simulink model "Demo_TISBeamShapes_cs"  (Simulink 7.6) (continued)
== system Demo_TISBeamShapes_cs == (continued)
      settings: DeleteFcn=PreScanBlockDeleteFcn;
      mask Help: eval('openHelp(222)')
      mask Display: image(imread('State.png'))
  STATE_Sphere_66 : Subsystem  Tag=State  Priority=0  mask(PreScan Sink Block: State){x0=20, y0=3, z0=4, Roll0=0, Pitch0=0, Yaw0=0, TimeStep=1/20}
      mask prompts: x0 "Ini x-position VEHICLE wrt GLOBAL axis system [m]"; y0 "Ini y-position VEHICLE wrt GLOBAL axis system [m]"; z0 "Ini z-position VEHICLE wrt GLOBAL axis system [m]"; Roll0 "Ini roll-angle BODY wrt VEHICLE axis system [deg]"; Pitch0 "Ini pitch-angle BODY wrt VEHICLE axis system [deg]"; Yaw0 "Ini yaw-angle VEHICLE wrt GLOBAL axis system [deg]"; TimeStep "Time Step [s]"
      mask help:
      | The Actor State contains a vector signal of size (9x1), containing the following signals:
      |
      | -  Position                  (x,y,z)                                     [m]
      | -  Velocity                  (vx,vy,vz)                               [m/s]
      | -  Angles                    (Roll, Pitch, Yaw)                   [rad]
      |
      | The Actor state is defined in the global axis system.
      | The state is superponed on the Initial position defined in the GUI.
      |
      | For more information, please click Help.
      settings: DeleteFcn=PreScanBlockDeleteFcn;
      mask Help: eval('openHelp(222)')
      mask Display: image(imread('State.png'))
  STATE_Sphere_67 : Subsystem  Tag=State  Priority=0  mask(PreScan Sink Block: State){x0=20, y0=3, z0=5, Roll0=0, Pitch0=0, Yaw0=0, TimeStep=1/20}
      mask prompts: x0 "Ini x-position VEHICLE wrt GLOBAL axis system [m]"; y0 "Ini y-position VEHICLE wrt GLOBAL axis system [m]"; z0 "Ini z-position VEHICLE wrt GLOBAL axis system [m]"; Roll0 "Ini roll-angle BODY wrt VEHICLE axis system [deg]"; Pitch0 "Ini pitch-angle BODY wrt VEHICLE axis system [deg]"; Yaw0 "Ini yaw-angle VEHICLE wrt GLOBAL axis system [deg]"; TimeStep "Time Step [s]"
      mask help:
      | The Actor State contains a vector signal of size (9x1), containing the following signals:
      |
      | -  Position                  (x,y,z)                                     [m]
      | -  Velocity                  (vx,vy,vz)                               [m/s]
      | -  Angles                    (Roll, Pitch, Yaw)                   [rad]
      |
      | The Actor state is defined in the global axis system.
      | The state is superponed on the Initial position defined in the GUI.
      |
      | For more information, please click Help.
      settings: DeleteFcn=PreScanBlockDeleteFcn;
      mask Help: eval('openHelp(222)')
      mask Display: image(imread('State.png'))
  STATE_Sphere_68 : Subsystem  Tag=State  Priority=0  mask(PreScan Sink Block: State){x0=20, y0=3, z0=6, Roll0=0, Pitch0=0, Yaw0=0, TimeStep=1/20}
      mask prompts: x0 "Ini x-position VEHICLE wrt GLOBAL axis system [m]"; y0 "Ini y-position VEHICLE wrt GLOBAL axis system [m]"; z0 "Ini z-position VEHICLE wrt GLOBAL axis system [m]"; Roll0 "Ini roll-angle BODY wrt VEHICLE axis system [deg]"; Pitch0 "Ini pitch-angle BODY wrt VEHICLE axis system [deg]"; Yaw0 "Ini yaw-angle VEHICLE wrt GLOBAL axis system [deg]"; TimeStep "Time Step [s]"
      mask help:
      | The Actor State contains a vector signal of size (9x1), containing the following signals:
      |
      | -  Position                  (x,y,z)                                     [m]
      | -  Velocity                  (vx,vy,vz)                               [m/s]
      | -  Angles                    (Roll, Pitch, Yaw)                   [rad]
      |
      | The Actor state is defined in the global axis system.
      | The state is superponed on the Initial position defined in the GUI.
      |
      | For more information, please click Help.
      settings: DeleteFcn=PreScanBlockDeleteFcn;
      mask Help: eval('openHelp(222)')
      mask Display: image(imread('State.png'))
  STATE_Sphere_69 : Subsystem  Tag=State  Priority=0  mask(PreScan Sink Block: State){x0=20, y0=3, z0=7, Roll0=0, Pitch0=0, Yaw0=0, TimeStep=1/20}
      mask prompts: x0 "Ini x-position VEHICLE wrt GLOBAL axis system [m]"; y0 "Ini y-position VEHICLE wrt GLOBAL axis system [m]"; z0 "Ini z-position VEHICLE wrt GLOBAL axis system [m]"; Roll0 "Ini roll-angle BODY wrt VEHICLE axis system [deg]"; Pitch0 "Ini pitch-angle BODY wrt VEHICLE axis system [deg]"; Yaw0 "Ini yaw-angle VEHICLE wrt GLOBAL axis system [deg]"; TimeStep "Time Step [s]"
      mask help:
      | The Actor State contains a vector signal of size (9x1), containing the following signals:
      |
      | -  Position                  (x,y,z)                                     [m]
      | -  Velocity                  (vx,vy,vz)                               [m/s]
      | -  Angles                    (Roll, Pitch, Yaw)                   [rad]
      |
      | The Actor state is defined in the global axis system.
      | The state is superponed on the Initial position defined in the GUI.
      |
      | For more information, please click Help.
      settings: DeleteFcn=PreScanBlockDeleteFcn;
      mask Help: eval('openHelp(222)')
      mask Display: image(imread('State.png'))
  STATE_Sphere_7 : Subsystem  Tag=State  Priority=0  mask(PreScan Sink Block: State){x0=20, y0=-4, z0=8, Roll0=0, Pitch0=0, Yaw0=0, TimeStep=1/20}
      mask prompts: x0 "Ini x-position VEHICLE wrt GLOBAL axis system [m]"; y0 "Ini y-position VEHICLE wrt GLOBAL axis system [m]"; z0 "Ini z-position VEHICLE wrt GLOBAL axis system [m]"; Roll0 "Ini roll-angle BODY wrt VEHICLE axis system [deg]"; Pitch0 "Ini pitch-angle BODY wrt VEHICLE axis system [deg]"; Yaw0 "Ini yaw-angle VEHICLE wrt GLOBAL axis system [deg]"; TimeStep "Time Step [s]"
      mask help:
      | The Actor State contains a vector signal of size (9x1), containing the following signals:
      |
      | -  Position                  (x,y,z)                                     [m]
      | -  Velocity                  (vx,vy,vz)                               [m/s]
      | -  Angles                    (Roll, Pitch, Yaw)                   [rad]
      |
      | The Actor state is defined in the global axis system.
      | The state is superponed on the Initial position defined in the GUI.
      |
      | For more information, please click Help.
      settings: DeleteFcn=PreScanBlockDeleteFcn;
      mask Help: eval('openHelp(222)')
      mask Display: image(imread('State.png'))
  STATE_Sphere_70 : Subsystem  Tag=State  Priority=0  mask(PreScan Sink Block: State){x0=20, y0=3, z0=8, Roll0=0, Pitch0=0, Yaw0=0, TimeStep=1/20}
      mask prompts: x0 "Ini x-position VEHICLE wrt GLOBAL axis system [m]"; y0 "Ini y-position VEHICLE wrt GLOBAL axis system [m]"; z0 "Ini z-position VEHICLE wrt GLOBAL axis system [m]"; Roll0 "Ini roll-angle BODY wrt VEHICLE axis system [deg]"; Pitch0 "Ini pitch-angle BODY wrt VEHICLE axis system [deg]"; Yaw0 "Ini yaw-angle VEHICLE wrt GLOBAL axis system [deg]"; TimeStep "Time Step [s]"
      mask help:
      | The Actor State contains a vector signal of size (9x1), containing the following signals:
      |
      | -  Position                  (x,y,z)                                     [m]
      | -  Velocity                  (vx,vy,vz)                               [m/s]
      | -  Angles                    (Roll, Pitch, Yaw)                   [rad]
      |
      | The Actor state is defined in the global axis system.
      | The state is superponed on the Initial position defined in the GUI.
      |
      | For more information, please click Help.
      settings: DeleteFcn=PreScanBlockDeleteFcn;
      mask Help: eval('openHelp(222)')
      mask Display: image(imread('State.png'))
  STATE_Sphere_71 : Subsystem  Tag=State  Priority=0  mask(PreScan Sink Block: State){x0=20, y0=3, z0=9, Roll0=0, Pitch0=0, Yaw0=0, TimeStep=1/20}
      mask prompts: x0 "Ini x-position VEHICLE wrt GLOBAL axis system [m]"; y0 "Ini y-position VEHICLE wrt GLOBAL axis system [m]"; z0 "Ini z-position VEHICLE wrt GLOBAL axis system [m]"; Roll0 "Ini roll-angle BODY wrt VEHICLE axis system [deg]"; Pitch0 "Ini pitch-angle BODY wrt VEHICLE axis system [deg]"; Yaw0 "Ini yaw-angle VEHICLE wrt GLOBAL axis system [deg]"; TimeStep "Time Step [s]"
      mask help:
      | The Actor State contains a vector signal of size (9x1), containing the following signals:
      |
      | -  Position                  (x,y,z)                                     [m]
      | -  Velocity                  (vx,vy,vz)                               [m/s]
      | -  Angles                    (Roll, Pitch, Yaw)                   [rad]
      |
      | The Actor state is defined in the global axis system.
      | The state is superponed on the Initial position defined in the GUI.
      |
      | For more information, please click Help.
      settings: DeleteFcn=PreScanBlockDeleteFcn;
      mask Help: eval('openHelp(222)')
      mask Display: image(imread('State.png'))
  STATE_Sphere_72 : Subsystem  Tag=State  Priority=0  mask(PreScan Sink Block: State){x0=20, y0=3, z0=10, Roll0=0, Pitch0=0, Yaw0=0, TimeStep=1/20}
      mask prompts: x0 "Ini x-position VEHICLE wrt GLOBAL axis system [m]"; y0 "Ini y-position VEHICLE wrt GLOBAL axis system [m]"; z0 "Ini z-position VEHICLE wrt GLOBAL axis system [m]"; Roll0 "Ini roll-angle BODY wrt VEHICLE axis system [deg]"; Pitch0 "Ini pitch-angle BODY wrt VEHICLE axis system [deg]"; Yaw0 "Ini yaw-angle VEHICLE wrt GLOBAL axis system [deg]"; TimeStep "Time Step [s]"
      mask help:
      | The Actor State contains a vector signal of size (9x1), containing the following signals:
      |
      | -  Position                  (x,y,z)                                     [m]
      | -  Velocity                  (vx,vy,vz)                               [m/s]
      | -  Angles                    (Roll, Pitch, Yaw)                   [rad]
      |
      | The Actor state is defined in the global axis system.
      | The state is superponed on the Initial position defined in the GUI.
      |
      | For more information, please click Help.
      settings: DeleteFcn=PreScanBlockDeleteFcn;
      mask Help: eval('openHelp(222)')
      mask Display: image(imread('State.png'))
  STATE_Sphere_73 : Subsystem  Tag=State  Priority=0  mask(PreScan Sink Block: State){x0=20, y0=4, z0=2, Roll0=0, Pitch0=0, Yaw0=0, TimeStep=1/20}
      mask prompts: x0 "Ini x-position VEHICLE wrt GLOBAL axis system [m]"; y0 "Ini y-position VEHICLE wrt GLOBAL axis system [m]"; z0 "Ini z-position VEHICLE wrt GLOBAL axis system [m]"; Roll0 "Ini roll-angle BODY wrt VEHICLE axis system [deg]"; Pitch0 "Ini pitch-angle BODY wrt VEHICLE axis system [deg]"; Yaw0 "Ini yaw-angle VEHICLE wrt GLOBAL axis system [deg]"; TimeStep "Time Step [s]"
      mask help:
      | The Actor State contains a vector signal of size (9x1), containing the following signals:
      |
      | -  Position                  (x,y,z)                                     [m]
      | -  Velocity                  (vx,vy,vz)                               [m/s]
      | -  Angles                    (Roll, Pitch, Yaw)                   [rad]
      |
      | The Actor state is defined in the global axis system.
      | The state is superponed on the Initial position defined in the GUI.
      |
      | For more information, please click Help.
      settings: DeleteFcn=PreScanBlockDeleteFcn;
      mask Help: eval('openHelp(222)')
      mask Display: image(imread('State.png'))
  STATE_Sphere_74 : Subsystem  Tag=State  Priority=0  mask(PreScan Sink Block: State){x0=20, y0=4, z0=3, Roll0=0, Pitch0=0, Yaw0=0, TimeStep=1/20}
      mask prompts: x0 "Ini x-position VEHICLE wrt GLOBAL axis system [m]"; y0 "Ini y-position VEHICLE wrt GLOBAL axis system [m]"; z0 "Ini z-position VEHICLE wrt GLOBAL axis system [m]"; Roll0 "Ini roll-angle BODY wrt VEHICLE axis system [deg]"; Pitch0 "Ini pitch-angle BODY wrt VEHICLE axis system [deg]"; Yaw0 "Ini yaw-angle VEHICLE wrt GLOBAL axis system [deg]"; TimeStep "Time Step [s]"
      mask help:
      | The Actor State contains a vector signal of size (9x1), containing the following signals:
      |
      | -  Position                  (x,y,z)                                     [m]
      | -  Velocity                  (vx,vy,vz)                               [m/s]
      | -  Angles                    (Roll, Pitch, Yaw)                   [rad]
      |
      | The Actor state is defined in the global axis system.
      | The state is superponed on the Initial position defined in the GUI.
      |
      | For more information, please click Help.
      settings: DeleteFcn=PreScanBlockDeleteFcn;
      mask Help: eval('openHelp(222)')
      mask Display: image(imread('State.png'))
  STATE_Sphere_75 : Subsystem  Tag=State  Priority=0  mask(PreScan Sink Block: State){x0=20, y0=4, z0=4, Roll0=0, Pitch0=0, Yaw0=0, TimeStep=1/20}
      mask prompts: x0 "Ini x-position VEHICLE wrt GLOBAL axis system [m]"; y0 "Ini y-position VEHICLE wrt GLOBAL axis system [m]"; z0 "Ini z-position VEHICLE wrt GLOBAL axis system [m]"; Roll0 "Ini roll-angle BODY wrt VEHICLE axis system [deg]"; Pitch0 "Ini pitch-angle BODY wrt VEHICLE axis system [deg]"; Yaw0 "Ini yaw-angle VEHICLE wrt GLOBAL axis system [deg]"; TimeStep "Time Step [s]"
      mask help:
      | The Actor State contains a vector signal of size (9x1), containing the following signals:
      |
      | -  Position                  (x,y,z)                                     [m]
      | -  Velocity                  (vx,vy,vz)                               [m/s]
      | -  Angles                    (Roll, Pitch, Yaw)                   [rad]
      |
      | The Actor state is defined in the global axis system.
      | The state is superponed on the Initial position defined in the GUI.
      |
      | For more information, please click Help.
      settings: DeleteFcn=PreScanBlockDeleteFcn;
      mask Help: eval('openHelp(222)')
      mask Display: image(imread('State.png'))
  STATE_Sphere_76 : Subsystem  Tag=State  Priority=0  mask(PreScan Sink Block: State){x0=20, y0=4, z0=5, Roll0=0, Pitch0=0, Yaw0=0, TimeStep=1/20}
      mask prompts: x0 "Ini x-position VEHICLE wrt GLOBAL axis system [m]"; y0 "Ini y-position VEHICLE wrt GLOBAL axis system [m]"; z0 "Ini z-position VEHICLE wrt GLOBAL axis system [m]"; Roll0 "Ini roll-angle BODY wrt VEHICLE axis system [deg]"; Pitch0 "Ini pitch-angle BODY wrt VEHICLE axis system [deg]"; Yaw0 "Ini yaw-angle VEHICLE wrt GLOBAL axis system [deg]"; TimeStep "Time Step [s]"
      mask help:
      | The Actor State contains a vector signal of size (9x1), containing the following signals:
      |
      | -  Position                  (x,y,z)                                     [m]
      | -  Velocity                  (vx,vy,vz)                               [m/s]
      | -  Angles                    (Roll, Pitch, Yaw)                   [rad]
      |
      | The Actor state is defined in the global axis system.
      | The state is superponed on the Initial position defined in the GUI.
      |
      | For more information, please click Help.
      settings: DeleteFcn=PreScanBlockDeleteFcn;
      mask Help: eval('openHelp(222)')
      mask Display: image(imread('State.png'))
  STATE_Sphere_77 : Subsystem  Tag=State  Priority=0  mask(PreScan Sink Block: State){x0=20, y0=4, z0=6, Roll0=0, Pitch0=0, Yaw0=0, TimeStep=1/20}
      mask prompts: x0 "Ini x-position VEHICLE wrt GLOBAL axis system [m]"; y0 "Ini y-position VEHICLE wrt GLOBAL axis system [m]"; z0 "Ini z-position VEHICLE wrt GLOBAL axis system [m]"; Roll0 "Ini roll-angle BODY wrt VEHICLE axis system [deg]"; Pitch0 "Ini pitch-angle BODY wrt VEHICLE axis system [deg]"; Yaw0 "Ini yaw-angle VEHICLE wrt GLOBAL axis system [deg]"; TimeStep "Time Step [s]"
      mask help:
      | The Actor State contains a vector signal of size (9x1), containing the following signals:
      |
      | -  Position                  (x,y,z)                                     [m]
      | -  Velocity                  (vx,vy,vz)                               [m/s]
      | -  Angles                    (Roll, Pitch, Yaw)                   [rad]
      |
      | The Actor state is defined in the global axis system.
      | The state is superponed on the Initial position defined in the GUI.
      |
      | For more information, please click Help.
      settings: DeleteFcn=PreScanBlockDeleteFcn;
      mask Help: eval('openHelp(222)')
      mask Display: image(imread('State.png'))
  STATE_Sphere_78 : Subsystem  Tag=State  Priority=0  mask(PreScan Sink Block: State){x0=20, y0=4, z0=7, Roll0=0, Pitch0=0, Yaw0=0, TimeStep=1/20}
      mask prompts: x0 "Ini x-position VEHICLE wrt GLOBAL axis system [m]"; y0 "Ini y-position VEHICLE wrt GLOBAL axis system [m]"; z0 "Ini z-position VEHICLE wrt GLOBAL axis system [m]"; Roll0 "Ini roll-angle BODY wrt VEHICLE axis system [deg]"; Pitch0 "Ini pitch-angle BODY wrt VEHICLE axis system [deg]"; Yaw0 "Ini yaw-angle VEHICLE wrt GLOBAL axis system [deg]"; TimeStep "Time Step [s]"
      mask help:
      | The Actor State contains a vector signal of size (9x1), containing the following signals:
      |
      | -  Position                  (x,y,z)                                     [m]
      | -  Velocity                  (vx,vy,vz)                               [m/s]
      | -  Angles                    (Roll, Pitch, Yaw)                   [rad]
      |
      | The Actor state is defined in the global axis system.
      | The state is superponed on the Initial position defined in the GUI.
      |
      | For more information, please click Help.
      settings: DeleteFcn=PreScanBlockDeleteFcn;
      mask Help: eval('openHelp(222)')
      mask Display: image(imread('State.png'))
  STATE_Sphere_79 : Subsystem  Tag=State  Priority=0  mask(PreScan Sink Block: State){x0=20, y0=4, z0=8, Roll0=0, Pitch0=0, Yaw0=0, TimeStep=1/20}
      mask prompts: x0 "Ini x-position VEHICLE wrt GLOBAL axis system [m]"; y0 "Ini y-position VEHICLE wrt GLOBAL axis system [m]"; z0 "Ini z-position VEHICLE wrt GLOBAL axis system [m]"; Roll0 "Ini roll-angle BODY wrt VEHICLE axis system [deg]"; Pitch0 "Ini pitch-angle BODY wrt VEHICLE axis system [deg]"; Yaw0 "Ini yaw-angle VEHICLE wrt GLOBAL axis system [deg]"; TimeStep "Time Step [s]"
      mask help:
      | The Actor State contains a vector signal of size (9x1), containing the following signals:
      |
      | -  Position                  (x,y,z)                                     [m]
      | -  Velocity                  (vx,vy,vz)                               [m/s]
      | -  Angles                    (Roll, Pitch, Yaw)                   [rad]
      |
      | The Actor state is defined in the global axis system.
      | The state is superponed on the Initial position defined in the GUI.
      |
      | For more information, please click Help.
      settings: DeleteFcn=PreScanBlockDeleteFcn;
      mask Help: eval('openHelp(222)')
      mask Display: image(imread('State.png'))
  STATE_Sphere_8 : Subsystem  Tag=State  Priority=0  mask(PreScan Sink Block: State){x0=20, y0=-4, z0=9, Roll0=0, Pitch0=0, Yaw0=0, TimeStep=1/20}
      mask prompts: x0 "Ini x-position VEHICLE wrt GLOBAL axis system [m]"; y0 "Ini y-position VEHICLE wrt GLOBAL axis system [m]"; z0 "Ini z-position VEHICLE wrt GLOBAL axis system [m]"; Roll0 "Ini roll-angle BODY wrt VEHICLE axis system [deg]"; Pitch0 "Ini pitch-angle BODY wrt VEHICLE axis system [deg]"; Yaw0 "Ini yaw-angle VEHICLE wrt GLOBAL axis system [deg]"; TimeStep "Time Step [s]"
      mask help:
      | The Actor State contains a vector signal of size (9x1), containing the following signals:
      |
      | -  Position                  (x,y,z)                                     [m]
      | -  Velocity                  (vx,vy,vz)                               [m/s]
      | -  Angles                    (Roll, Pitch, Yaw)                   [rad]
      |
      | The Actor state is defined in the global axis system.
      | The state is superponed on the Initial position defined in the GUI.
      |
      | For more information, please click Help.
      settings: DeleteFcn=PreScanBlockDeleteFcn;
      mask Help: eval('openHelp(222)')
      mask Display: image(imread('State.png'))
  STATE_Sphere_80 : Subsystem  Tag=State  Priority=0  mask(PreScan Sink Block: State){x0=20, y0=4, z0=9, Roll0=0, Pitch0=0, Yaw0=0, TimeStep=1/20}
      mask prompts: x0 "Ini x-position VEHICLE wrt GLOBAL axis system [m]"; y0 "Ini y-position VEHICLE wrt GLOBAL axis system [m]"; z0 "Ini z-position VEHICLE wrt GLOBAL axis system [m]"; Roll0 "Ini roll-angle BODY wrt VEHICLE axis system [deg]"; Pitch0 "Ini pitch-angle BODY wrt VEHICLE axis system [deg]"; Yaw0 "Ini yaw-angle VEHICLE wrt GLOBAL axis system [deg]"; TimeStep "Time Step [s]"
      mask help:
      | The Actor State contains a vector signal of size (9x1), containing the following signals:
      |
      | -  Position                  (x,y,z)                                     [m]
      | -  Velocity                  (vx,vy,vz)                               [m/s]
      | -  Angles                    (Roll, Pitch, Yaw)                   [rad]
      |
      | The Actor state is defined in the global axis system.
      | The state is superponed on the Initial position defined in the GUI.
      |
      | For more information, please click Help.
      settings: DeleteFcn=PreScanBlockDeleteFcn;
      mask Help: eval('openHelp(222)')
      mask Display: image(imread('State.png'))
  STATE_Sphere_81 : Subsystem  Tag=State  Priority=0  mask(PreScan Sink Block: State){x0=20, y0=4, z0=10, Roll0=0, Pitch0=0, Yaw0=0, TimeStep=1/20}
      mask prompts: x0 "Ini x-position VEHICLE wrt GLOBAL axis system [m]"; y0 "Ini y-position VEHICLE wrt GLOBAL axis system [m]"; z0 "Ini z-position VEHICLE wrt GLOBAL axis system [m]"; Roll0 "Ini roll-angle BODY wrt VEHICLE axis system [deg]"; Pitch0 "Ini pitch-angle BODY wrt VEHICLE axis system [deg]"; Yaw0 "Ini yaw-angle VEHICLE wrt GLOBAL axis system [deg]"; TimeStep "Time Step [s]"
      mask help:
      | The Actor State contains a vector signal of size (9x1), containing the following signals:
      |
      | -  Position                  (x,y,z)                                     [m]
      | -  Velocity                  (vx,vy,vz)                               [m/s]
      | -  Angles                    (Roll, Pitch, Yaw)                   [rad]
      |
      | The Actor state is defined in the global axis system.
      | The state is superponed on the Initial position defined in the GUI.
      |
      | For more information, please click Help.
      settings: DeleteFcn=PreScanBlockDeleteFcn;
      mask Help: eval('openHelp(222)')
      mask Display: image(imread('State.png'))
  STATE_Sphere_9 : Subsystem  Tag=State  Priority=0  mask(PreScan Sink Block: State){x0=20, y0=-4, z0=10, Roll0=0, Pitch0=0, Yaw0=0, TimeStep=1/20}
      mask prompts: x0 "Ini x-position VEHICLE wrt GLOBAL axis system [m]"; y0 "Ini y-position VEHICLE wrt GLOBAL axis system [m]"; z0 "Ini z-position VEHICLE wrt GLOBAL axis system [m]"; Roll0 "Ini roll-angle BODY wrt VEHICLE axis system [deg]"; Pitch0 "Ini pitch-angle BODY wrt VEHICLE axis system [deg]"; Yaw0 "Ini yaw-angle VEHICLE wrt GLOBAL axis system [deg]"; TimeStep "Time Step [s]"
      mask help:
      | The Actor State contains a vector signal of size (9x1), containing the following signals:
      |
      | -  Position                  (x,y,z)                                     [m]
      | -  Velocity                  (vx,vy,vz)                               [m/s]
      | -  Angles                    (Roll, Pitch, Yaw)                   [rad]
      |
      | The Actor state is defined in the global axis system.
      | The state is superponed on the Initial position defined in the GUI.
      |
      | For more information, please click Help.
      settings: DeleteFcn=PreScanBlockDeleteFcn;
      mask Help: eval('openHelp(222)')
      mask Display: image(imread('State.png'))
  SetActuators : Subsystem  Tag=SetActuators  Priority=1  mask(PreScan Simulation Core Block){TimeStep=1/20, NrOfActuators=1260}
      mask prompts: TimeStep "Timestep [s]"; NrOfActuators "Number of actuator values"
      mask help:
      | Please do not remove this block from the PreScan compilation sheet,
      | as it contains essential configuration parameters for a PreScan simulation.
      settings: DeleteFcn=PreScanBlockDeleteFcn;
  SimCoreConfiguration : M-S-Function  Tag=SimCoreConfiguration  Description=CAD8B8374CE53CE7DF3567A6B8CC3B00F170F6ABFE8D6F1F6CD897A2C413C5A6  FunctionName=SimCoreBlock  Parameters=ExperimentXML PortsXML SimulationCore HostName CheckStart EnableLog RfR EnableGPUAccel ShowComputationTime  mask(PreScan Simulation Core Configurator){SimulationCore=NUTS, HostName=0, CheckStart=0, prescanversion='7.4.0', EnableLog=off, RfR=0, EnableGPUAccel=off, ShowComputationTime=off}
      mask prompts: SimulationCore "Simulation Core"; HostName "Simulation Host"; CheckStart "Show START Dialog"; prescanversion "PreScan Version"; EnableLog "Create TA Log"; RfR "Record for Replay"; EnableGPUAccel "Enable GPU Acceleration"; ShowComputationTime "Show ComputationTime Scope"
      mask help:
      | Please do not remove this block from the PreScan compilation sheet,
      | as it contains essential configuration parameter for a PreScan simulation.
      mask init:
      | model = bdroot;
      | ExperimentXML = [model(1:end-3) '.pex'];
      | PortsXML = [model '_ports.pcd'];
      settings: DeleteFcn=PreScanBlockDeleteFcn;  ModelCloseFcn=assignin('base',['model_closed_' bdroot],true);
      mask Help: eval('openHelp(812)')
      mask Display: image(imread('prescanlogo.ico'))
  SimCoreInfo : Subsystem  Tag=SimCoreInfo
  Sphere_1 : Subsystem  Tag=WorldObjectSubSystem  InitFcn=UniqueId = '1001';
      mask Display: image(imread('Sphere.jpg'));color('white');text(0,0,' ID=1001\n')
  Sphere_10 : Subsystem  Tag=WorldObjectSubSystem  InitFcn=UniqueId = '1010';
      mask Display: image(imread('Sphere.jpg'));color('white');text(0,0,' ID=1010\n')
  Sphere_11 : Subsystem  Tag=WorldObjectSubSystem  InitFcn=UniqueId = '1011';
      mask Display: image(imread('Sphere.jpg'));color('white');text(0,0,' ID=1011\n')
  Sphere_12 : Subsystem  Tag=WorldObjectSubSystem  InitFcn=UniqueId = '1012';
      mask Display: image(imread('Sphere.jpg'));color('white');text(0,0,' ID=1012\n')
  Sphere_13 : Subsystem  Tag=WorldObjectSubSystem  InitFcn=UniqueId = '1013';
      mask Display: image(imread('Sphere.jpg'));color('white');text(0,0,' ID=1013\n')
  Sphere_14 : Subsystem  Tag=WorldObjectSubSystem  InitFcn=UniqueId = '1014';
      mask Display: image(imread('Sphere.jpg'));color('white');text(0,0,' ID=1014\n')
  Sphere_15 : Subsystem  Tag=WorldObjectSubSystem  InitFcn=UniqueId = '1015';
      mask Display: image(imread('Sphere.jpg'));color('white');text(0,0,' ID=1015\n')
  Sphere_16 : Subsystem  Tag=WorldObjectSubSystem  InitFcn=UniqueId = '1016';
      mask Display: image(imread('Sphere.jpg'));color('white');text(0,0,' ID=1016\n')
  Sphere_17 : Subsystem  Tag=WorldObjectSubSystem  InitFcn=UniqueId = '1017';
      mask Display: image(imread('Sphere.jpg'));color('white');text(0,0,' ID=1017\n')
  Sphere_18 : Subsystem  Tag=WorldObjectSubSystem  InitFcn=UniqueId = '1018';
      mask Display: image(imread('Sphere.jpg'));color('white');text(0,0,' ID=1018\n')
  Sphere_19 : Subsystem  Tag=WorldObjectSubSystem  InitFcn=UniqueId = '1019';
      mask Display: image(imread('Sphere.jpg'));color('white');text(0,0,' ID=1019\n')
  Sphere_2 : Subsystem  Tag=WorldObjectSubSystem  InitFcn=UniqueId = '1002';
      mask Display: image(imread('Sphere.jpg'));color('white');text(0,0,' ID=1002\n')
  Sphere_20 : Subsystem  Tag=WorldObjectSubSystem  InitFcn=UniqueId = '1020';
      mask Display: image(imread('Sphere.jpg'));color('white');text(0,0,' ID=1020\n')
  Sphere_21 : Subsystem  Tag=WorldObjectSubSystem  InitFcn=UniqueId = '1021';
      mask Display: image(imread('Sphere.jpg'));color('white');text(0,0,' ID=1021\n')
  Sphere_22 : Subsystem  Tag=WorldObjectSubSystem  InitFcn=UniqueId = '1022';
      mask Display: image(imread('Sphere.jpg'));color('white');text(0,0,' ID=1022\n')
  Sphere_23 : Subsystem  Tag=WorldObjectSubSystem  InitFcn=UniqueId = '1023';
      mask Display: image(imread('Sphere.jpg'));color('white');text(0,0,' ID=1023\n')
  Sphere_24 : Subsystem  Tag=WorldObjectSubSystem  InitFcn=UniqueId = '1024';
      mask Display: image(imread('Sphere.jpg'));color('white');text(0,0,' ID=1024\n')
  Sphere_25 : Subsystem  Tag=WorldObjectSubSystem  InitFcn=UniqueId = '1025';
      mask Display: image(imread('Sphere.jpg'));color('white');text(0,0,' ID=1025\n')
  Sphere_26 : Subsystem  Tag=WorldObjectSubSystem  InitFcn=UniqueId = '1026';
      mask Display: image(imread('Sphere.jpg'));color('white');text(0,0,' ID=1026\n')
  Sphere_27 : Subsystem  Tag=WorldObjectSubSystem  InitFcn=UniqueId = '1027';
      mask Display: image(imread('Sphere.jpg'));color('white');text(0,0,' ID=1027\n')
  Sphere_28 : Subsystem  Tag=WorldObjectSubSystem  InitFcn=UniqueId = '1028';
      mask Display: image(imread('Sphere.jpg'));color('white');text(0,0,' ID=1028\n')
  Sphere_29 : Subsystem  Tag=WorldObjectSubSystem  InitFcn=UniqueId = '1029';
      mask Display: image(imread('Sphere.jpg'));color('white');text(0,0,' ID=1029\n')
  Sphere_3 : Subsystem  Tag=WorldObjectSubSystem  InitFcn=UniqueId = '1003';
      mask Display: image(imread('Sphere.jpg'));color('white');text(0,0,' ID=1003\n')
  Sphere_30 : Subsystem  Tag=WorldObjectSubSystem  InitFcn=UniqueId = '1030';
      mask Display: image(imread('Sphere.jpg'));color('white');text(0,0,' ID=1030\n')
  Sphere_31 : Subsystem  Tag=WorldObjectSubSystem  InitFcn=UniqueId = '1031';
      mask Display: image(imread('Sphere.jpg'));color('white');text(0,0,' ID=1031\n')
  Sphere_32 : Subsystem  Tag=WorldObjectSubSystem  InitFcn=UniqueId = '1032';
      mask Display: image(imread('Sphere.jpg'));color('white');text(0,0,' ID=1032\n')
  Sphere_33 : Subsystem  Tag=WorldObjectSubSystem  InitFcn=UniqueId = '1033';
      mask Display: image(imread('Sphere.jpg'));color('white');text(0,0,' ID=1033\n')
  Sphere_34 : Subsystem  Tag=WorldObjectSubSystem  InitFcn=UniqueId = '1034';
      mask Display: image(imread('Sphere.jpg'));color('white');text(0,0,' ID=1034\n')
  Sphere_35 : Subsystem  Tag=WorldObjectSubSystem  InitFcn=UniqueId = '1035';
      mask Display: image(imread('Sphere.jpg'));color('white');text(0,0,' ID=1035\n')
  Sphere_36 : Subsystem  Tag=WorldObjectSubSystem  InitFcn=UniqueId = '1036';
      mask Display: image(imread('Sphere.jpg'));color('white');text(0,0,' ID=1036\n')
  Sphere_37 : Subsystem  Tag=WorldObjectSubSystem  InitFcn=UniqueId = '1037';
      mask Display: image(imread('Sphere.jpg'));color('white');text(0,0,' ID=1037\n')
  Sphere_38 : Subsystem  Tag=WorldObjectSubSystem  InitFcn=UniqueId = '1038';
      mask Display: image(imread('Sphere.jpg'));color('white');text(0,0,' ID=1038\n')
  Sphere_39 : Subsystem  Tag=WorldObjectSubSystem  InitFcn=UniqueId = '1039';
      mask Display: image(imread('Sphere.jpg'));color('white');text(0,0,' ID=1039\n')
  Sphere_4 : Subsystem  Tag=WorldObjectSubSystem  InitFcn=UniqueId = '1004';
      mask Display: image(imread('Sphere.jpg'));color('white');text(0,0,' ID=1004\n')
  Sphere_40 : Subsystem  Tag=WorldObjectSubSystem  InitFcn=UniqueId = '1040';
      mask Display: image(imread('Sphere.jpg'));color('white');text(0,0,' ID=1040\n')
  Sphere_41 : Subsystem  Tag=WorldObjectSubSystem  InitFcn=UniqueId = '1041';
      mask Display: image(imread('Sphere.jpg'));color('white');text(0,0,' ID=1041\n')
  Sphere_42 : Subsystem  Tag=WorldObjectSubSystem  InitFcn=UniqueId = '1042';
      mask Display: image(imread('Sphere.jpg'));color('white');text(0,0,' ID=1042\n')
  Sphere_43 : Subsystem  Tag=WorldObjectSubSystem  InitFcn=UniqueId = '1043';
      mask Display: image(imread('Sphere.jpg'));color('white');text(0,0,' ID=1043\n')
  Sphere_44 : Subsystem  Tag=WorldObjectSubSystem  InitFcn=UniqueId = '1044';
      mask Display: image(imread('Sphere.jpg'));color('white');text(0,0,' ID=1044\n')
  Sphere_45 : Subsystem  Tag=WorldObjectSubSystem  InitFcn=UniqueId = '1045';
      mask Display: image(imread('Sphere.jpg'));color('white');text(0,0,' ID=1045\n')
  Sphere_46 : Subsystem  Tag=WorldObjectSubSystem  InitFcn=UniqueId = '1046';
      mask Display: image(imread('Sphere.jpg'));color('white');text(0,0,' ID=1046\n')
  Sphere_47 : Subsystem  Tag=WorldObjectSubSystem  InitFcn=UniqueId = '1047';
      mask Display: image(imread('Sphere.jpg'));color('white');text(0,0,' ID=1047\n')
  Sphere_48 : Subsystem  Tag=WorldObjectSubSystem  InitFcn=UniqueId = '1048';
      mask Display: image(imread('Sphere.jpg'));color('white');text(0,0,' ID=1048\n')
  Sphere_49 : Subsystem  Tag=WorldObjectSubSystem  InitFcn=UniqueId = '1049';
      mask Display: image(imread('Sphere.jpg'));color('white');text(0,0,' ID=1049\n')
  Sphere_5 : Subsystem  Tag=WorldObjectSubSystem  InitFcn=UniqueId = '1005';
      mask Display: image(imread('Sphere.jpg'));color('white');text(0,0,' ID=1005\n')
  Sphere_50 : Subsystem  Tag=WorldObjectSubSystem  InitFcn=UniqueId = '1050';
      mask Display: image(imread('Sphere.jpg'));color('white');text(0,0,' ID=1050\n')
  Sphere_51 : Subsystem  Tag=WorldObjectSubSystem  InitFcn=UniqueId = '1051';
      mask Display: image(imread('Sphere.jpg'));color('white');text(0,0,' ID=1051\n')
  Sphere_52 : Subsystem  Tag=WorldObjectSubSystem  InitFcn=UniqueId = '1052';
      mask Display: image(imread('Sphere.jpg'));color('white');text(0,0,' ID=1052\n')
  Sphere_53 : Subsystem  Tag=WorldObjectSubSystem  InitFcn=UniqueId = '1053';
      mask Display: image(imread('Sphere.jpg'));color('white');text(0,0,' ID=1053\n')
  Sphere_54 : Subsystem  Tag=WorldObjectSubSystem  InitFcn=UniqueId = '1054';
      mask Display: image(imread('Sphere.jpg'));color('white');text(0,0,' ID=1054\n')
  Sphere_55 : Subsystem  Tag=WorldObjectSubSystem  InitFcn=UniqueId = '1055';
      mask Display: image(imread('Sphere.jpg'));color('white');text(0,0,' ID=1055\n')
  Sphere_56 : Subsystem  Tag=WorldObjectSubSystem  InitFcn=UniqueId = '1056';
      mask Display: image(imread('Sphere.jpg'));color('white');text(0,0,' ID=1056\n')
  Sphere_57 : Subsystem  Tag=WorldObjectSubSystem  InitFcn=UniqueId = '1057';
      mask Display: image(imread('Sphere.jpg'));color('white');text(0,0,' ID=1057\n')
  Sphere_58 : Subsystem  Tag=WorldObjectSubSystem  InitFcn=UniqueId = '1058';
      mask Display: image(imread('Sphere.jpg'));color('white');text(0,0,' ID=1058\n')
  Sphere_59 : Subsystem  Tag=WorldObjectSubSystem  InitFcn=UniqueId = '1059';
      mask Display: image(imread('Sphere.jpg'));color('white');text(0,0,' ID=1059\n')
  Sphere_6 : Subsystem  Tag=WorldObjectSubSystem  InitFcn=UniqueId = '1006';
      mask Display: image(imread('Sphere.jpg'));color('white');text(0,0,' ID=1006\n')
  Sphere_60 : Subsystem  Tag=WorldObjectSubSystem  InitFcn=UniqueId = '1060';
      mask Display: image(imread('Sphere.jpg'));color('white');text(0,0,' ID=1060\n')
  Sphere_61 : Subsystem  Tag=WorldObjectSubSystem  InitFcn=UniqueId = '1061';
      mask Display: image(imread('Sphere.jpg'));color('white');text(0,0,' ID=1061\n')
  Sphere_62 : Subsystem  Tag=WorldObjectSubSystem  InitFcn=UniqueId = '1062';
      mask Display: image(imread('Sphere.jpg'));color('white');text(0,0,' ID=1062\n')
  Sphere_63 : Subsystem  Tag=WorldObjectSubSystem  InitFcn=UniqueId = '1063';
      mask Display: image(imread('Sphere.jpg'));color('white');text(0,0,' ID=1063\n')
  Sphere_64 : Subsystem  Tag=WorldObjectSubSystem  InitFcn=UniqueId = '1064';
      mask Display: image(imread('Sphere.jpg'));color('white');text(0,0,' ID=1064\n')
  Sphere_65 : Subsystem  Tag=WorldObjectSubSystem  InitFcn=UniqueId = '1065';
      mask Display: image(imread('Sphere.jpg'));color('white');text(0,0,' ID=1065\n')
  Sphere_66 : Subsystem  Tag=WorldObjectSubSystem  InitFcn=UniqueId = '1066';
      mask Display: image(imread('Sphere.jpg'));color('white');text(0,0,' ID=1066\n')
  Sphere_67 : Subsystem  Tag=WorldObjectSubSystem  InitFcn=UniqueId = '1067';
      mask Display: image(imread('Sphere.jpg'));color('white');text(0,0,' ID=1067\n')
  Sphere_68 : Subsystem  Tag=WorldObjectSubSystem  InitFcn=UniqueId = '1068';
      mask Display: image(imread('Sphere.jpg'));color('white');text(0,0,' ID=1068\n')
  Sphere_69 : Subsystem  Tag=WorldObjectSubSystem  InitFcn=UniqueId = '1069';
      mask Display: image(imread('Sphere.jpg'));color('white');text(0,0,' ID=1069\n')
  Sphere_7 : Subsystem  Tag=WorldObjectSubSystem  InitFcn=UniqueId = '1007';
      mask Display: image(imread('Sphere.jpg'));color('white');text(0,0,' ID=1007\n')
  Sphere_70 : Subsystem  Tag=WorldObjectSubSystem  InitFcn=UniqueId = '1070';
      mask Display: image(imread('Sphere.jpg'));color('white');text(0,0,' ID=1070\n')
  Sphere_71 : Subsystem  Tag=WorldObjectSubSystem  InitFcn=UniqueId = '1071';
      mask Display: image(imread('Sphere.jpg'));color('white');text(0,0,' ID=1071\n')
  Sphere_72 : Subsystem  Tag=WorldObjectSubSystem  InitFcn=UniqueId = '1072';
      mask Display: image(imread('Sphere.jpg'));color('white');text(0,0,' ID=1072\n')
  Sphere_73 : Subsystem  Tag=WorldObjectSubSystem  InitFcn=UniqueId = '1073';
      mask Display: image(imread('Sphere.jpg'));color('white');text(0,0,' ID=1073\n')
  Sphere_74 : Subsystem  Tag=WorldObjectSubSystem  InitFcn=UniqueId = '1074';
      mask Display: image(imread('Sphere.jpg'));color('white');text(0,0,' ID=1074\n')
  Sphere_75 : Subsystem  Tag=WorldObjectSubSystem  InitFcn=UniqueId = '1075';
      mask Display: image(imread('Sphere.jpg'));color('white');text(0,0,' ID=1075\n')
  Sphere_76 : Subsystem  Tag=WorldObjectSubSystem  InitFcn=UniqueId = '1076';
      mask Display: image(imread('Sphere.jpg'));color('white');text(0,0,' ID=1076\n')
  Sphere_77 : Subsystem  Tag=WorldObjectSubSystem  InitFcn=UniqueId = '1077';
      mask Display: image(imread('Sphere.jpg'));color('white');text(0,0,' ID=1077\n')
  Sphere_78 : Subsystem  Tag=WorldObjectSubSystem  InitFcn=UniqueId = '1078';
      mask Display: image(imread('Sphere.jpg'));color('white');text(0,0,' ID=1078\n')
  Sphere_79 : Subsystem  Tag=WorldObjectSubSystem  InitFcn=UniqueId = '1079';
      mask Display: image(imread('Sphere.jpg'));color('white');text(0,0,' ID=1079\n')
  Sphere_8 : Subsystem  Tag=WorldObjectSubSystem  InitFcn=UniqueId = '1008';
      mask Display: image(imread('Sphere.jpg'));color('white');text(0,0,' ID=1008\n')
  Sphere_80 : Subsystem  Tag=WorldObjectSubSystem  InitFcn=UniqueId = '1080';
      mask Display: image(imread('Sphere.jpg'));color('white');text(0,0,' ID=1080\n')
  Sphere_81 : Subsystem  Tag=WorldObjectSubSystem  InitFcn=UniqueId = '1081';
      mask Display: image(imread('Sphere.jpg'));color('white');text(0,0,' ID=1081\n')
  Sphere_9 : Subsystem  Tag=WorldObjectSubSystem  InitFcn=UniqueId = '1009';
      mask Display: image(imread('Sphere.jpg'));color('white');text(0,0,' ID=1009\n')
  TIS_1 : S-Function  Priority=3  LoadFcn=load('PrescanScannerSensorData.mat');load('PrescanScannerSensorMessage.mat')  FunctionName=sfun_TISSensor  Parameters=timestepMask maxInputMessages maxOutputMessages sensorID detectableObjects  EnableBusSupport=off  mask{timestepMask=1/20, maxInputMessages=0, maxOutputMessages=405, sensorID=12, detectableObjects=81}
      mask prompts: timestepMask "MATLAB timestep:"; maxInputMessages "Max input messages:"; maxOutputMessages "Max output messages:"; sensorID "Sensor ID:"; detectableObjects "Max detectable objects:"
      mask help: TIS Sensor I/O
      mask Display: image(imread('TIS.png'))
  wires:
  Sphere_1/1(STATE_Sphere_1) -> STATE_Sphere_1/1(State)
  SELF_Sphere_1/1(U) -> Sphere_1/1(SELF_Sphere_1)
  Sphere_2/1(STATE_Sphere_2) -> STATE_Sphere_2/1(State)
  SELF_Sphere_2/1(U) -> Sphere_2/1(SELF_Sphere_2)
  Sphere_3/1(STATE_Sphere_3) -> STATE_Sphere_3/1(State)
  SELF_Sphere_3/1(U) -> Sphere_3/1(SELF_Sphere_3)
  Sphere_4/1(STATE_Sphere_4) -> STATE_Sphere_4/1(State)
  SELF_Sphere_4/1(U) -> Sphere_4/1(SELF_Sphere_4)
  Sphere_5/1(STATE_Sphere_5) -> STATE_Sphere_5/1(State)
  SELF_Sphere_5/1(U) -> Sphere_5/1(SELF_Sphere_5)
  Sphere_6/1(STATE_Sphere_6) -> STATE_Sphere_6/1(State)
  SELF_Sphere_6/1(U) -> Sphere_6/1(SELF_Sphere_6)
  Sphere_7/1(STATE_Sphere_7) -> STATE_Sphere_7/1(State)
  SELF_Sphere_7/1(U) -> Sphere_7/1(SELF_Sphere_7)
  Sphere_8/1(STATE_Sphere_8) -> STATE_Sphere_8/1(State)
  SELF_Sphere_8/1(U) -> Sphere_8/1(SELF_Sphere_8)
  Sphere_9/1(STATE_Sphere_9) -> STATE_Sphere_9/1(State)
  SELF_Sphere_9/1(U) -> Sphere_9/1(SELF_Sphere_9)
  Sphere_10/1(STATE_Sphere_10) -> STATE_Sphere_10/1(State)
  SELF_Sphere_10/1(U) -> Sphere_10/1(SELF_Sphere_10)
  Sphere_11/1(STATE_Sphere_11) -> STATE_Sphere_11/1(State)
  SELF_Sphere_11/1(U) -> Sphere_11/1(SELF_Sphere_11)
  Sphere_12/1(STATE_Sphere_12) -> STATE_Sphere_12/1(State)
  SELF_Sphere_12/1(U) -> Sphere_12/1(SELF_Sphere_12)
  Sphere_13/1(STATE_Sphere_13) -> STATE_Sphere_13/1(State)
  SELF_Sphere_13/1(U) -> Sphere_13/1(SELF_Sphere_13)
  Sphere_14/1(STATE_Sphere_14) -> STATE_Sphere_14/1(State)
  SELF_Sphere_14/1(U) -> Sphere_14/1(SELF_Sphere_14)
  Sphere_15/1(STATE_Sphere_15) -> STATE_Sphere_15/1(State)
  SELF_Sphere_15/1(U) -> Sphere_15/1(SELF_Sphere_15)
  Sphere_16/1(STATE_Sphere_16) -> STATE_Sphere_16/1(State)
  SELF_Sphere_16/1(U) -> Sphere_16/1(SELF_Sphere_16)
  Sphere_17/1(STATE_Sphere_17) -> STATE_Sphere_17/1(State)
  SELF_Sphere_17/1(U) -> Sphere_17/1(SELF_Sphere_17)
  Sphere_18/1(STATE_Sphere_18) -> STATE_Sphere_18/1(State)
  SELF_Sphere_18/1(U) -> Sphere_18/1(SELF_Sphere_18)
  Sphere_19/1(STATE_Sphere_19) -> STATE_Sphere_19/1(State)
  SELF_Sphere_19/1(U) -> Sphere_19/1(SELF_Sphere_19)
  Sphere_20/1(STATE_Sphere_20) -> STATE_Sphere_20/1(State)
  SELF_Sphere_20/1(U) -> Sphere_20/1(SELF_Sphere_20)
  Sphere_21/1(STATE_Sphere_21) -> STATE_Sphere_21/1(State)
  SELF_Sphere_21/1(U) -> Sphere_21/1(SELF_Sphere_21)
  Sphere_22/1(STATE_Sphere_22) -> STATE_Sphere_22/1(State)
  SELF_Sphere_22/1(U) -> Sphere_22/1(SELF_Sphere_22)
  Sphere_23/1(STATE_Sphere_23) -> STATE_Sphere_23/1(State)
  SELF_Sphere_23/1(U) -> Sphere_23/1(SELF_Sphere_23)
  Sphere_24/1(STATE_Sphere_24) -> STATE_Sphere_24/1(State)
  SELF_Sphere_24/1(U) -> Sphere_24/1(SELF_Sphere_24)
  Sphere_25/1(STATE_Sphere_25) -> STATE_Sphere_25/1(State)
  SELF_Sphere_25/1(U) -> Sphere_25/1(SELF_Sphere_25)
  Sphere_26/1(STATE_Sphere_26) -> STATE_Sphere_26/1(State)
  SELF_Sphere_26/1(U) -> Sphere_26/1(SELF_Sphere_26)
  Sphere_27/1(STATE_Sphere_27) -> STATE_Sphere_27/1(State)
  SELF_Sphere_27/1(U) -> Sphere_27/1(SELF_Sphere_27)
  Sphere_28/1(STATE_Sphere_28) -> STATE_Sphere_28/1(State)
  SELF_Sphere_28/1(U) -> Sphere_28/1(SELF_Sphere_28)
  Sphere_29/1(STATE_Sphere_29) -> STATE_Sphere_29/1(State)
  SELF_Sphere_29/1(U) -> Sphere_29/1(SELF_Sphere_29)
  Sphere_30/1(STATE_Sphere_30) -> STATE_Sphere_30/1(State)
  SELF_Sphere_30/1(U) -> Sphere_30/1(SELF_Sphere_30)
  Sphere_31/1(STATE_Sphere_31) -> STATE_Sphere_31/1(State)
  SELF_Sphere_31/1(U) -> Sphere_31/1(SELF_Sphere_31)
  Sphere_32/1(STATE_Sphere_32) -> STATE_Sphere_32/1(State)
  SELF_Sphere_32/1(U) -> Sphere_32/1(SELF_Sphere_32)
  Sphere_33/1(STATE_Sphere_33) -> STATE_Sphere_33/1(State)
  SELF_Sphere_33/1(U) -> Sphere_33/1(SELF_Sphere_33)
  Sphere_34/1(STATE_Sphere_34) -> STATE_Sphere_34/1(State)
  SELF_Sphere_34/1(U) -> Sphere_34/1(SELF_Sphere_34)
  Sphere_35/1(STATE_Sphere_35) -> STATE_Sphere_35/1(State)
  SELF_Sphere_35/1(U) -> Sphere_35/1(SELF_Sphere_35)
  Sphere_36/1(STATE_Sphere_36) -> STATE_Sphere_36/1(State)
  SELF_Sphere_36/1(U) -> Sphere_36/1(SELF_Sphere_36)
  Sphere_37/1(STATE_Sphere_37) -> STATE_Sphere_37/1(State)
  SELF_Sphere_37/1(U) -> Sphere_37/1(SELF_Sphere_37)
  Sphere_38/1(STATE_Sphere_38) -> STATE_Sphere_38/1(State)
  SELF_Sphere_38/1(U) -> Sphere_38/1(SELF_Sphere_38)
  Sphere_39/1(STATE_Sphere_39) -> STATE_Sphere_39/1(State)
  SELF_Sphere_39/1(U) -> Sphere_39/1(SELF_Sphere_39)
  Sphere_40/1(STATE_Sphere_40) -> STATE_Sphere_40/1(State)
  SELF_Sphere_40/1(U) -> Sphere_40/1(SELF_Sphere_40)
  Sphere_41/1(STATE_Sphere_41) -> STATE_Sphere_41/1(State)
  SELF_Sphere_41/1(U) -> Sphere_41/1(SELF_Sphere_41)
  Sphere_42/1(STATE_Sphere_42) -> STATE_Sphere_42/1(State)
  SELF_Sphere_42/1(U) -> Sphere_42/1(SELF_Sphere_42)
  Sphere_43/1(STATE_Sphere_43) -> STATE_Sphere_43/1(State)
  SELF_Sphere_43/1(U) -> Sphere_43/1(SELF_Sphere_43)
  Sphere_44/1(STATE_Sphere_44) -> STATE_Sphere_44/1(State)
  SELF_Sphere_44/1(U) -> Sphere_44/1(SELF_Sphere_44)
  Sphere_45/1(STATE_Sphere_45) -> STATE_Sphere_45/1(State)
  SELF_Sphere_45/1(U) -> Sphere_45/1(SELF_Sphere_45)
  Sphere_46/1(STATE_Sphere_46) -> STATE_Sphere_46/1(State)
  SELF_Sphere_46/1(U) -> Sphere_46/1(SELF_Sphere_46)
  Sphere_47/1(STATE_Sphere_47) -> STATE_Sphere_47/1(State)
  SELF_Sphere_47/1(U) -> Sphere_47/1(SELF_Sphere_47)
  Sphere_48/1(STATE_Sphere_48) -> STATE_Sphere_48/1(State)
  SELF_Sphere_48/1(U) -> Sphere_48/1(SELF_Sphere_48)
  Sphere_49/1(STATE_Sphere_49) -> STATE_Sphere_49/1(State)
  SELF_Sphere_49/1(U) -> Sphere_49/1(SELF_Sphere_49)
  Sphere_50/1(STATE_Sphere_50) -> STATE_Sphere_50/1(State)
  SELF_Sphere_50/1(U) -> Sphere_50/1(SELF_Sphere_50)
  Sphere_51/1(STATE_Sphere_51) -> STATE_Sphere_51/1(State)
  SELF_Sphere_51/1(U) -> Sphere_51/1(SELF_Sphere_51)
  Sphere_52/1(STATE_Sphere_52) -> STATE_Sphere_52/1(State)
  SELF_Sphere_52/1(U) -> Sphere_52/1(SELF_Sphere_52)
  Sphere_53/1(STATE_Sphere_53) -> STATE_Sphere_53/1(State)
  SELF_Sphere_53/1(U) -> Sphere_53/1(SELF_Sphere_53)
  Sphere_54/1(STATE_Sphere_54) -> STATE_Sphere_54/1(State)
  SELF_Sphere_54/1(U) -> Sphere_54/1(SELF_Sphere_54)
  Sphere_55/1(STATE_Sphere_55) -> STATE_Sphere_55/1(State)
  SELF_Sphere_55/1(U) -> Sphere_55/1(SELF_Sphere_55)
  Sphere_56/1(STATE_Sphere_56) -> STATE_Sphere_56/1(State)
  SELF_Sphere_56/1(U) -> Sphere_56/1(SELF_Sphere_56)
  Sphere_57/1(STATE_Sphere_57) -> STATE_Sphere_57/1(State)
  SELF_Sphere_57/1(U) -> Sphere_57/1(SELF_Sphere_57)
  Sphere_58/1(STATE_Sphere_58) -> STATE_Sphere_58/1(State)
  SELF_Sphere_58/1(U) -> Sphere_58/1(SELF_Sphere_58)
  Sphere_59/1(STATE_Sphere_59) -> STATE_Sphere_59/1(State)
  SELF_Sphere_59/1(U) -> Sphere_59/1(SELF_Sphere_59)
  Sphere_60/1(STATE_Sphere_60) -> STATE_Sphere_60/1(State)
  SELF_Sphere_60/1(U) -> Sphere_60/1(SELF_Sphere_60)
  Sphere_61/1(STATE_Sphere_61) -> STATE_Sphere_61/1(State)
  SELF_Sphere_61/1(U) -> Sphere_61/1(SELF_Sphere_61)
  Sphere_62/1(STATE_Sphere_62) -> STATE_Sphere_62/1(State)
  SELF_Sphere_62/1(U) -> Sphere_62/1(SELF_Sphere_62)
  Sphere_63/1(STATE_Sphere_63) -> STATE_Sphere_63/1(State)
  SELF_Sphere_63/1(U) -> Sphere_63/1(SELF_Sphere_63)
  Sphere_64/1(STATE_Sphere_64) -> STATE_Sphere_64/1(State)
  SELF_Sphere_64/1(U) -> Sphere_64/1(SELF_Sphere_64)
  Sphere_65/1(STATE_Sphere_65) -> STATE_Sphere_65/1(State)
  SELF_Sphere_65/1(U) -> Sphere_65/1(SELF_Sphere_65)
  Sphere_66/1(STATE_Sphere_66) -> STATE_Sphere_66/1(State)
  SELF_Sphere_66/1(U) -> Sphere_66/1(SELF_Sphere_66)
  Sphere_67/1(STATE_Sphere_67) -> STATE_Sphere_67/1(State)
  SELF_Sphere_67/1(U) -> Sphere_67/1(SELF_Sphere_67)
  Sphere_68/1(STATE_Sphere_68) -> STATE_Sphere_68/1(State)
  SELF_Sphere_68/1(U) -> Sphere_68/1(SELF_Sphere_68)
  Sphere_69/1(STATE_Sphere_69) -> STATE_Sphere_69/1(State)
  SELF_Sphere_69/1(U) -> Sphere_69/1(SELF_Sphere_69)
  Sphere_70/1(STATE_Sphere_70) -> STATE_Sphere_70/1(State)
  SELF_Sphere_70/1(U) -> Sphere_70/1(SELF_Sphere_70)
  Sphere_71/1(STATE_Sphere_71) -> STATE_Sphere_71/1(State)
  SELF_Sphere_71/1(U) -> Sphere_71/1(SELF_Sphere_71)
  Sphere_72/1(STATE_Sphere_72) -> STATE_Sphere_72/1(State)
  SELF_Sphere_72/1(U) -> Sphere_72/1(SELF_Sphere_72)
  Sphere_73/1(STATE_Sphere_73) -> STATE_Sphere_73/1(State)
  SELF_Sphere_73/1(U) -> Sphere_73/1(SELF_Sphere_73)
  Sphere_74/1(STATE_Sphere_74) -> STATE_Sphere_74/1(State)
  SELF_Sphere_74/1(U) -> Sphere_74/1(SELF_Sphere_74)
  Sphere_75/1(STATE_Sphere_75) -> STATE_Sphere_75/1(State)
  SELF_Sphere_75/1(U) -> Sphere_75/1(SELF_Sphere_75)
  Sphere_76/1(STATE_Sphere_76) -> STATE_Sphere_76/1(State)
  SELF_Sphere_76/1(U) -> Sphere_76/1(SELF_Sphere_76)
  Sphere_77/1(STATE_Sphere_77) -> STATE_Sphere_77/1(State)
  SELF_Sphere_77/1(U) -> Sphere_77/1(SELF_Sphere_77)
  Sphere_78/1(STATE_Sphere_78) -> STATE_Sphere_78/1(State)
  SELF_Sphere_78/1(U) -> Sphere_78/1(SELF_Sphere_78)
  Sphere_79/1(STATE_Sphere_79) -> STATE_Sphere_79/1(State)
  SELF_Sphere_79/1(U) -> Sphere_79/1(SELF_Sphere_79)
  Sphere_80/1(STATE_Sphere_80) -> STATE_Sphere_80/1(State)
  SELF_Sphere_80/1(U) -> Sphere_80/1(SELF_Sphere_80)
  Sphere_81/1(STATE_Sphere_81) -> STATE_Sphere_81/1(State)
  SELF_Sphere_81/1(U) -> Sphere_81/1(SELF_Sphere_81)
  Cone_1/1(STATE_Cone_1) -> STATE_Cone_1/1(State)
  SELF_Cone_1/1(U) -> Cone_1/1(SELF_Cone_1)
  TIS_1/1 -> Cone_1/2(TIS_1)
  Cylinder_1/1(STATE_Cylinder_1) -> STATE_Cylinder_1/1(State)
  SELF_Cylinder_1/1(U) -> Cylinder_1/1(SELF_Cylinder_1)
  Cylinder_2/1(STATE_Cylinder_2) -> STATE_Cylinder_2/1(State)
  SELF_Cylinder_2/1(U) -> Cylinder_2/1(SELF_Cylinder_2)
  SetActuators/1(dummy) -> GetSensors/1(dummy)
